simulink model "TosAeb"  (R2021a)
settings: Solver=FixedStepDiscrete  StartTime=0.0  StopTime=inf  FixedStep=0.01  RelTol=1e-3
undefined symbols (not defined in the file or in its folder): ACCModSts, AccSts1, AEB_ObjectMovingOrientation, AEB_ObjectMovingState, CN_BTCActive, CN_CurrentGearR, CN_CurrentGearR_DHT, CN_PTCActive, CN_VDCActive, CN_VSC_EspOff, CN_VSC_FCW_AEB_Error, CN_VSC_FCW_AEB_NoDispay, CN_VSC_FCW_AEB_PerfmDgdtn, CN_VSC_FCW_AEB_TempUnAvlbl, CN_VSC_VehStandstill, CNaccelAvoidMax, CNaccelAvoidMin, CNbrakePedalPress, CNcomfortStrLeftAccelMax, CNcomfortStrLeftAccelMin, CNcomfortStrRightAccelMax, CNcomfortStrRightAccelMin, CNDrvDoorOpen, CNDrvModeEco, CNDrvModeNorm, CNDrvModeOther, CNDrvModeSport, CNDrvSBRreminder, CNNull, CNobjInvalidID, CNPassDoorOpen, CNPassSBRreminder, CNPEBSAccelNegInf, CNPEBSAccelNull, CNPEBSTimeInf, CNPEBSTimeNull, CNSTOPMAX, CNSTOPNULL, CNsubjWidth, CNSwtOFF, CNSwtON, CNTTCMAX, CNTTCNULL, CNVehConfigAEBCycNone, CNVehConfigAEBNone, CNVehConfigAEBPedNone, CNVehConfigFCWNone, CNVehConfigJANone, CollisionSeverity, FALSE, FCWSnvty, JACycObjCalcInfoDefaultParams, JAPedObjCalcInfoDefaultParams, JAVehObjCalcInfoDefaultParams, latCycObjCalcInfoDefaultParams, latPedObjCalcInfoDefaultParams, longObjCalcInfoDefaultParams, ModSts, movingStatus, ObjectType ... (+1112)
libraries: simulink x3031, AEBSFunctionLib x50, Ta_Library x33, AEBSStateMachineLib x23, Mylibrary x12, simulink_extras x7, ObjSelect_lib x3, dspstat3 x2
(part 67 of 102)

== system TosAeb/AEB_Objsel/TA_Processor/AEB_ObjSelect/TargetInfoCheck/Data_precess/TA_EffectCalculate/ValideateObj/ObjPos_Calculate/ObjPos_Calculateelse/InPath_Cal/DetermineInPath ==
  ALat : Inport
  VLat : Inport  Port=2
  PathEstimn : Inport  Port=3
  VLgtRel : Inport  Port=4
  PosnLat : Inport  Port=5
  PosnLgt : Inport  Port=6
  ObjWidth : Inport  Port=7
  HostWidth : Inport  Port=8
  Abs : Abs
  Add1 : Sum  Inputs=+-
  Add2 : Sum  Inputs=+-
  Add3 : Sum
  Add4 : Sum
  Compare To Zero : Subsystem  mask(Compare To Zero){relop=<, OutDataTypeStr=boolean, ZeroCross=on}
      mask prompts: relop "Simulink:masks:Operator_MP"; OutDataTypeStr "Simulink:masks:OutputDataType_MP"; ZeroCross "Simulink:masks:EnableZerocrossingDetection_MP"
      mask help: Simulink:masks:CompareToZero_MD
      mask init: same as TosAeb/AEB_Objsel/TA_Processor/AEB_ObjSelect/TargetInfoCheck/Data_precess/TA_EffectCalculate/Obj_FilterFlgCalculate/Long_ObjFilter/TTR_Calculate/CalculateTTRAndTTM_else/ModifyTTRAtSameSpeedAndAcceleration/Compare To Zero
      mask TypeOptions relop: == | ~= | < | <= | >= | >
      mask TypeOptions OutDataTypeStr: Simulink:masks:uint8 | Simulink:masks:boolean
      mask Callback ZeroCross: sl('zeroCrossEnable', gcbh);
      mask Display: disp([relop,' 0'])
  Constant : Constant  Value=1.8  OutDataTypeStr=single
  Constant2 : Constant  Value=single(0.5)
  Divide4 : Product  Inputs=**
  LimitedSafeDivide : Subsystem
  OppositeSign : Gain  Gain=single(-1)
  PredictHostLateralPositionAtTTR : Subsystem
  PredictObjectLateralPositionAtTTR : Subsystem
  Terminator{1..4} (4 alike) : Terminator
  InPath : Outport
  wires:
  PathEstimn/1 -> PredictHostLateralPositionAtTTR/2(PahEstimn)
  VLgtRel/1 -> OppositeSign/1
  Constant2/1 -> Divide4/2
  OppositeSign/1 -> LimitedSafeDivide/2(Denominator)
  PosnLat/1 -> PredictObjectLateralPositionAtTTR/1(Posn)
  PredictHostLateralPositionAtTTR/3(PredHostHeading) -> Terminator1/1
  Compare To Zero/1(y) -> InPath/1  "InPath"
  PredictObjectLateralPositionAtTTR/2(VPred) -> Terminator3/1
  PredictHostLateralPositionAtTTR/2(PredHostPosnLat) -> Add1/2  "PosnLatPredHost"
  Add4/1 -> Divide4/1  "ObjectAndHostWidth"
  Add1/1 -> Abs/1  "PosnLatPredRel"
  Abs/1 -> Add2/1  "LateralDistance"
  PredictObjectLateralPositionAtTTR/3(APred) -> Terminator4/1
  Divide4/1 -> Add2/2  "HalfObjectAndHostWidth"
  Add2/1 -> Compare To Zero/1(u)  "LatDistanceEdgeToEdge"
  PredictObjectLateralPositionAtTTR/1(PosnPred) -> Add1/1  "PosnLatPredObj"
  PredictHostLateralPositionAtTTR/1(PredHostPosnLgt) -> Terminator2/1
  LimitedSafeDivide/1(LimitedQuotient) -> PredictHostLateralPositionAtTTR/1(PredictionTime), PredictObjectLateralPositionAtTTR/4(Ti)  "SimpleTTR"
  VLat/1 -> PredictObjectLateralPositionAtTTR/2(V)
  ALat/1 -> PredictObjectLateralPositionAtTTR/3(A)
  PosnLgt/1 -> LimitedSafeDivide/1(Numerator)
  ObjWidth/1 -> Add4/1
  HostWidth/1 -> Add3/1
  Add3/1 -> Add4/2  "ObjectAndHostWidth"
  Constant/1 -> Add3/2

== system TosAeb/AEB_Objsel/TA_Processor/AEB_ObjSelect/TargetInfoCheck/Data_precess/TA_EffectCalculate/ValideateObj/ValidObj_PreProcess/If Action Subsystem1/CheckPosition/Subsystem ==
  ObsDx : Inport
  If : If
  Merge : Merge
  dxCalc_if : Subsystem  TreatAsAtomicUnit=on
  dxcalc : Subsystem  TreatAsAtomicUnit=on
  Obsdx : Outport
  wires:
  ObsDx/1 -> [A], If/1
  If/1 -> dxCalc_if/Action
  If/2 -> dxcalc/Action
  [A] -> dxCalc_if/1(ObsDx)
  dxCalc_if/1(Obsdx) -> Merge/1
  dxcalc/1(Obsdx) -> Merge/2
  Merge/1 -> Obsdx/1

== system TosAeb/AEB_Objsel/TA_Processor/AEB_ObjSelect/TargetInfoCheck/Data_precess/TA_EffectCalculate/ValideateObj/ValidObj_PreProcess/If Action Subsystem1/CheckType/Bicycle ==
  TA_ObsType : Inport
  Constant8 : Constant  Value=5  OutDataTypeStr=uint8
  Relational Operator8 : RelationalOperator  Operator===  OutDataTypeStr=boolean  RndMeth=Simplest
  Bicycle_Flg : Outport
  wires:
  TA_ObsType/1 -> Relational Operator8/1
  Constant8/1 -> Relational Operator8/2
  Relational Operator8/1 -> Bicycle_Flg/1

== system TosAeb/AEB_Objsel/TA_Processor/AEB_ObjSelect/TargetInfoCheck/Data_precess/TA_EffectCalculate/ValideateObj/ValidObj_PreProcess/If Action Subsystem1/CheckType/Bus ==
  TA_ObsType : Inport
  Constant6 : Constant  OutDataTypeStr=uint8
  Relational Operator6 : RelationalOperator  Operator===  OutDataTypeStr=boolean  RndMeth=Simplest
  Bus_Flg : Outport
  wires:
  TA_ObsType/1 -> Relational Operator6/1
  Constant6/1 -> Relational Operator6/2
  Relational Operator6/1 -> Bus_Flg/1

== system TosAeb/AEB_Objsel/TA_Processor/AEB_ObjSelect/TargetInfoCheck/Data_precess/TA_EffectCalculate/ValideateObj/ValidObj_PreProcess/If Action Subsystem1/CheckType/Car ==
  TA_ObsType : Inport
  Constant3 : Constant  Value=2  OutDataTypeStr=uint8
  Relational Operator3 : RelationalOperator  Operator===  OutDataTypeStr=boolean  RndMeth=Simplest
  Car_Flg : Outport
  wires:
  TA_ObsType/1 -> Relational Operator3/1
  Constant3/1 -> Relational Operator3/2
  Relational Operator3/1 -> Car_Flg/1

== system TosAeb/AEB_Objsel/TA_Processor/AEB_ObjSelect/TargetInfoCheck/Data_precess/TA_EffectCalculate/ValideateObj/ValidObj_PreProcess/If Action Subsystem1/CheckType/Invalid ==
  TA_ObjType : Inport
  Constant1 : Constant  Value=7  OutDataTypeStr=uint8
  Constant8 : Constant  Value=0  OutDataTypeStr=uint8
  Logical Operator : Logic  Operator=OR  AllPortsSameDT=off  OutDataTypeStr=boolean
  Logical Operator1 : Logic  Operator=NOT  AllPortsSameDT=off  OutDataTypeStr=boolean
  Relational Operator1 : RelationalOperator  Operator===  OutDataTypeStr=boolean  RndMeth=Simplest
  Relational Operator8 : RelationalOperator  Operator===  OutDataTypeStr=boolean  RndMeth=Simplest
  Invalid_Flg : Outport
  wires:
  TA_ObjType/1 -> Relational Operator1/1, Relational Operator8/1
  Constant8/1 -> Relational Operator8/2
  Constant1/1 -> Relational Operator1/2
  Relational Operator8/1 -> Logical Operator/1
  Relational Operator1/1 -> Logical Operator/2
  Logical Operator/1 -> Logical Operator1/1
  Logical Operator1/1 -> Invalid_Flg/1

== system TosAeb/AEB_Objsel/TA_Processor/AEB_ObjSelect/TargetInfoCheck/Data_precess/TA_EffectCalculate/ValideateObj/ValidObj_PreProcess/If Action Subsystem1/CheckType/MotorBike ==
  TA_ObsType : Inport
  Constant7 : Constant  Value=4  OutDataTypeStr=uint8
  Relational Operator7 : RelationalOperator  Operator===  OutDataTypeStr=boolean  RndMeth=Simplest
  MotorBike : Outport
  wires:
  Constant7/1 -> Relational Operator7/2
  Relational Operator7/1 -> MotorBike/1
  TA_ObsType/1 -> Relational Operator7/1

== system TosAeb/AEB_Objsel/TA_Processor/AEB_ObjSelect/TargetInfoCheck/Data_precess/TA_EffectCalculate/ValideateObj/ValidObj_PreProcess/If Action Subsystem1/CheckType/Pedestrain ==
  TA_ObjType : Inport
  Constant8 : Constant  Value=6  OutDataTypeStr=uint8
  Relational Operator8 : RelationalOperator  Operator===  OutDataTypeStr=boolean  RndMeth=Simplest
  Pedestrian_Flg : Outport
  wires:
  Constant8/1 -> Relational Operator8/2
  TA_ObjType/1 -> Relational Operator8/1
  Relational Operator8/1 -> Pedestrian_Flg/1

== system TosAeb/AEB_Objsel/TA_Processor/AEB_ObjSelect/TargetInfoCheck/Data_precess/TA_EffectCalculate/ValideateObj/ValidObj_PreProcess/If Action Subsystem1/CheckType/Truck ==
  TA_ObsType : Inport
  Constant2 : Constant  Value=3  OutDataTypeStr=uint8
  Relational Operator2 : RelationalOperator  Operator===  OutDataTypeStr=boolean  RndMeth=Simplest
  Truck_Flg : Outport
  wires:
  Constant2/1 -> Relational Operator2/2
  TA_ObsType/1 -> Relational Operator2/1
  Relational Operator2/1 -> Truck_Flg/1

== system TosAeb/AEB_Objsel/TA_Processor/PreProcessor/ObjectPrecessor/TAObjInfoCalc/For Each Subsystem/Info_Check1/BasicInfo_check/AccCorrect/Acc_XY/Subsystem ==
  ObsAccY_s : Inport
  Obj_vy : Inport  Port=2
  Add : Sum
  Add2 : Sum  Inputs=+-
  Compare To Constant : simulink/Logic and Bit Operations/Compare To Constant  relop=>=  const=2  OutDataTypeStr=boolean
  Constant : Constant  Value=0.1  OutDataTypeStr=single
  Constant4 : Constant  Value=P_TA_ObjAyLowPassFactor
  Delay : Delay
  Divide : Product  Inputs=*/
  LowPassFilter : Ta_Library/LowPassFilter  SystemSampleTime=-1  Latency=0  AutoFrameSizeCalculation=off
  Switch : Switch  Criteria=u2 ~= 0
  ObsAy_Correct : Outport
  wires:
  Divide/1 -> Add2/2
  ObsAccY_s/1 -> [ObsAccY_s], Add2/1, LowPassFilter/2
  Add2/1 -> Compare To Constant/1
  Obj_vy/1 -> Delay/1, Add/1
  Delay/1 -> Add/2
  Add/1 -> Divide/1
  Constant/1 -> Divide/2
  Compare To Constant/1 -> Switch/2
  Constant4/1 -> LowPassFilter/1
  LowPassFilter/1 -> Switch/1
  [ObsAccY_s] -> Switch/3
  Switch/1 -> ObsAy_Correct/1

== system TosAeb/AEB_Objsel/TA_Processor/PreProcessor/ObjectPrecessor/TAObjInfoCalc/For Each Subsystem/Info_Check1/BasicInfo_check/AccCorrect/Acc_XY/Subsystem1 ==
  ObsAccX_s : Inport
  Obj_vX : Inport  Port=2
  Add : Sum
  Add2 : Sum  Inputs=+-
  Compare To Constant : simulink/Logic and Bit Operations/Compare To Constant  relop=>=  const=2  OutDataTypeStr=boolean
  Constant : Constant  Value=0.1  OutDataTypeStr=single
  Constant4 : Constant  Value=P_TA_ObjAxLowPassFactor
  Delay : Delay
  Divide : Product  Inputs=*/
  LowPassFilter : Ta_Library/LowPassFilter  SystemSampleTime=-1  Latency=0  AutoFrameSizeCalculation=off
  Switch : Switch  Criteria=u2 ~= 0
  ObsAX_Correct : Outport
  wires:
  Switch/1 -> ObsAX_Correct/1
  [ObsAccY_s] -> Switch/3
  LowPassFilter/1 -> Switch/1
  Constant4/1 -> LowPassFilter/1
  Compare To Constant/1 -> Switch/2
  Constant/1 -> Divide/2
  Add/1 -> Divide/1
  Delay/1 -> Add/2
  Obj_vX/1 -> Add/1, Delay/1
  Add2/1 -> Compare To Constant/1
  ObsAccX_s/1 -> LowPassFilter/2, Add2/1, [ObsAccY_s]
  Divide/1 -> Add2/2

== system TosAeb/AEB_Objsel/TA_Processor/PreProcessor/ObjectPrecessor/TAObjInfoCalc/For Each Subsystem/Info_Check1/BasicInfo_check/DxCorrect/dx_charge/Dx_Flag ==
  Fus_ObjVx_Single : Inport
  Veh_AEBFunState : Inport  Port=2
  Fus_ObjDxStd : Inport  Port=3
  Fus_ObjDx_Single : Inport  Port=4
  Compare To Constant : simulink/Logic and Bit Operations/Compare To Constant  relop=>=  const=P_TA_ObjDxRateVarianceThredValue  OutDataTypeStr=boolean
  Compare To Constant1 : simulink/Logic and Bit Operations/Compare To Constant  relop=>=  const=P_TA_ObjDxSTDThredValue  OutDataTypeStr=boolean
  Data Type Conversion : DataTypeConversion
  Logical Operator : Logic  Operator=OR  Inputs=3  AllPortsSameDT=off  OutDataTypeStr=boolean
  Logical Operator1 : Logic  AllPortsSameDT=off  OutDataTypeStr=boolean
  RateVariance_Cal : Ta_Library/RateVariance_Cal  SystemSampleTime=-1  Latency=0  AutoFrameSizeCalculation=off
  dx_AebState : Subsystem
  dx_compare : Subsystem
  Dx_CorrectFlg : Outport
  wires:
  RateVariance_Cal/1 -> Compare To Constant/1
  Fus_ObjDx_Single/1 -> RateVariance_Cal/1, dx_compare/1(Fus_ObjDx_Single)
  dx_AebState/1(AEB_State) -> Logical Operator1/2
  Compare To Constant/1 -> Logical Operator/1
  Veh_AEBFunState/1 -> dx_AebState/1(Veh_AEBFunState)
  Logical Operator/1 -> Logical Operator1/1
  dx_compare/1(DisLong_flg) -> Logical Operator/2
  Fus_ObjVx_Single/1 -> dx_compare/2(Fus_ObjVx_Single)
  Logical Operator1/1 -> Data Type Conversion/1
  Data Type Conversion/1 -> Dx_CorrectFlg/1
  Compare To Constant1/1 -> Logical Operator/3
  Fus_ObjDxStd/1 -> Compare To Constant1/1

== system TosAeb/AEB_Objsel/TA_Processor/PreProcessor/ObjectPrecessor/TAObjInfoCalc/For Each Subsystem/Info_Check1/BasicInfo_check/DxCorrect/dx_charge/dx_change ==
  Dx_CorrectFlg : Inport
  Fus_ObjDx_Single : Inport  Port=2
  Constant : Constant  Value=P_TA_ObjDxLowPassFactor
  Constant1 : Constant  Value=0  OutDataTypeStr=boolean
  LowPassFilter : Ta_Library/LowPassFilter  SystemSampleTime=-1  Latency=0  AutoFrameSizeCalculation=off
  Switch : Switch  Criteria=u2 ~= 0
  Terminator_1 : Terminator
  Fus_ObjDx : Outport
  wires:
  LowPassFilter/1 -> Switch/1
  Switch/1 -> Fus_ObjDx/1
  Constant/1 -> LowPassFilter/1
  Constant1/1 -> Switch/2
  Dx_CorrectFlg/1 -> Terminator_1/1
  Fus_ObjDx_Single/1 -> Switch/3, LowPassFilter/2

== system TosAeb/AEB_Objsel/TA_Processor/PreProcessor/ObjectPrecessor/TAObjInfoCalc/For Each Subsystem/Info_Check1/BasicInfo_check/DyCorrect/dy_charge/Subsystem2 ==
  Fus_ObjVy_Single : Inport
  Veh_AEBFunState : Inport  Port=2
  Fus_ObsDyStd : Inport  Port=3
  Fus_ObjDy_Single : Inport  Port=4
  Compare To Constant : simulink/Logic and Bit Operations/Compare To Constant  relop=>=  const=P_TA_ObjDyRateVarianceThredValue  OutDataTypeStr=boolean
  Compare To Constant1 : simulink/Logic and Bit Operations/Compare To Constant  relop=>=  const=P_TA_ObjDySTDThredValue  OutDataTypeStr=boolean
  Data Type Conversion : DataTypeConversion
  Logical Operator : Logic  Operator=OR  Inputs=3  AllPortsSameDT=off  OutDataTypeStr=boolean
  Logical Operator1 : Logic  AllPortsSameDT=off  OutDataTypeStr=boolean
  RateVariance_Cal : Ta_Library/RateVariance_Cal  SystemSampleTime=-1  Latency=0  AutoFrameSizeCalculation=off
  Subsystem1 : Subsystem
  charge : Subsystem
  Dy_CorrectFlg : Outport
  wires:
  Data Type Conversion/1 -> Dy_CorrectFlg/1
  Logical Operator1/1 -> Data Type Conversion/1
  Fus_ObjVy_Single/1 -> charge/2(Fus_ObjVy_Single)
  charge/1(DisLat_flg) -> Logical Operator/2
  Logical Operator/1 -> Logical Operator1/1
  Veh_AEBFunState/1 -> Subsystem1/1(Veh_AEBFunState)
  Compare To Constant/1 -> Logical Operator/1
  Subsystem1/1(AEB_State) -> Logical Operator1/2
  Fus_ObjDy_Single/1 -> charge/1(Fus_ObjDy_Single), RateVariance_Cal/1
  RateVariance_Cal/1 -> Compare To Constant/1
  Compare To Constant1/1 -> Logical Operator/3
  Fus_ObsDyStd/1 -> Compare To Constant1/1

== system TosAeb/AEB_Objsel/TA_Processor/PreProcessor/ObjectPrecessor/TAObjInfoCalc/For Each Subsystem/Info_Check1/BasicInfo_check/DyCorrect/dy_charge/dy_change ==
  Dy_CorrectFlg : Inport
  Fus_ObjDy_Single : Inport  Port=2
  Constant : Constant  Value=P_TA_ObjDyLowPassFactor
  Constant1 : Constant  Value=0  OutDataTypeStr=boolean
  LowPassFilter : Ta_Library/LowPassFilter  SystemSampleTime=-1  Latency=0  AutoFrameSizeCalculation=off
  Scope : Scope  NumInputPorts=3
  Switch : Switch  Criteria=u2 ~= 0
  Terminator : Terminator
  Fus_ObjDy : Outport
  wires:
  Switch/1 -> Scope/3, Fus_ObjDy/1
  LowPassFilter/1 -> Switch/1, Scope/2
  Fus_ObjDy_Single/1 -> LowPassFilter/2, Scope/1, Switch/3
  Constant/1 -> LowPassFilter/1
  Constant1/1 -> Switch/2
  Dy_CorrectFlg/1 -> Terminator/1

== system TosAeb/AEB_Objsel/TA_Processor/PreProcessor/ObjectPrecessor/TAObjInfoCalc/For Each Subsystem/Info_Check1/BasicInfo_check/HeadAngle/If Action Subsystem1/HeadAngle_c ==
  ID_Switch : Inport
  ObsAngle_s : Inport  Port=2
  ObsAngleSTD_s : Inport  Port=3
  Obj_VxCr : Inport  Port=4
  Obj_VyCr : Inport  Port=5
  Add : Sum  Inputs=+-
  Add1 : Sum
  Compare To Constant : simulink/Logic and Bit Operations/Compare To Constant  relop=<  const=P_TA_ObjHeadAngleDiffThred  OutDataTypeStr=boolean
  Compare To Constant1 : simulink/Logic and Bit Operations/Compare To Constant  relop=<  const=P_TA_ObjHeadAngleSTDThredValue  OutDataTypeStr=boolean
  Constant : Constant  Value=P_TA_ObjHeadAngleLowPassFactor
  Constant1 : Constant  Value=0.5  OutDataTypeStr=single
  Constant2 : Constant  Value=0  OutDataTypeStr=single
  Logical Operator : Logic  AllPortsSameDT=off  OutDataTypeStr=boolean
  LowPassFilter : Ta_Library/LowPassFilter  SystemSampleTime=-1  Latency=0  AutoFrameSizeCalculation=off
  Product : Product
  Subsystem2 : Subsystem
  Switch : Switch  Criteria=u2 > Threshold
  Switch1 : Switch  Criteria=u2 > Threshold
  Fus_ObjHeadAngle : Outport
  wires:
  Obj_VxCr/1 -> Subsystem2/2(Obj_Vx)
  Obj_VyCr/1 -> Subsystem2/1(Obj_vy)
  Subsystem2/1(Obj_Head) -> Add1/1, Add/2
  ObsAngle_s/1 -> [ObsAngle_s], Add/1
  Add/1 -> Compare To Constant/1
  ObsAngleSTD_s/1 -> Compare To Constant1/1
  Compare To Constant1/1 -> Logical Operator/1
  Compare To Constant/1 -> Logical Operator/2
  Logical Operator/1 -> Switch/2
  [ObsAngle_s] -> Switch/1
  [ObsAngle_s] -> Add1/2
  Add1/1 -> Product/1
  Constant/1 -> LowPassFilter/1
  Constant1/1 -> Product/2
  Product/1 -> LowPassFilter/2
  LowPassFilter/1 -> Switch/3
  Switch/1 -> Switch1/3
  ID_Switch/1 -> Switch1/2
  Switch1/1 -> Fus_ObjHeadAngle/1
  Constant2/1 -> Switch1/1

== system TosAeb/AEB_Objsel/TA_Processor/PreProcessor/ObjectPrecessor/TAObjInfoCalc/For Each Subsystem/Info_Check1/BasicInfo_check/HeadAngle/If Action Subsystem1/Type_class ==
  Fus_Type : Inport
  Compare To Constant : simulink/Logic and Bit Operations/Compare To Constant  relop=<  const=1  OutDataTypeStr=boolean
  Compare To Constant1 : simulink/Logic and Bit Operations/Compare To Constant  relop=>  const=10  OutDataTypeStr=boolean
  Data Type Conversion : DataTypeConversion
  Logical Operator : Logic  Operator=OR  AllPortsSameDT=off  OutDataTypeStr=boolean
  tyoe_enable : Outport
  wires:
  Fus_Type/1 -> Compare To Constant1/1, Compare To Constant/1
  Compare To Constant/1 -> Logical Operator/1
  Compare To Constant1/1 -> Logical Operator/2
  Logical Operator/1 -> Data Type Conversion/1
  Data Type Conversion/1 -> tyoe_enable/1

== system TosAeb/AEB_Objsel/TA_Processor/PreProcessor/ObjectPrecessor/TAObjInfoCalc/For Each Subsystem/Info_Check1/BasicInfo_check/QuaCorrect/quality_recrectionelse/Quality_else ==
  Fus_TargetQuality : Inport
  dy_flg : Inport  Port=2
  dx_flg : Inport  Port=3
  vx_flg : Inport  Port=4
  vy_flg : Inport  Port=5
  Action Port : ActionPort
  Factor_Cal : Subsystem
  Quality_Out : Subsystem
  Fus_ObjQuality : Outport
  wires:
  vx_flg/1 -> Factor_Cal/3(vx_flg)
  Quality_Out/1(Fus_ObjQuality) -> Fus_ObjQuality/1
  dy_flg/1 -> Factor_Cal/1(dy_flg)
  Factor_Cal/1(factor) -> Quality_Out/2(factor)
  dx_flg/1 -> Factor_Cal/2(dx_flg)
  vy_flg/1 -> Factor_Cal/4(vy_flg)
  Fus_TargetQuality/1 -> Quality_Out/1(Fus_TargetQuality)

== system TosAeb/AEB_Objsel/TA_Processor/PreProcessor/ObjectPrecessor/TAObjInfoCalc/For Each Subsystem/Info_Check1/BasicInfo_check/QuaCorrect/quality_recrectionelse/Quality_if ==
  Fus_TargetQuality : Inport
  Action Port : ActionPort
  Signal Conversion : SignalConversion  OverrideOpt=off
  Fus_ObjQuality : Outport
  wires:
  Signal Conversion/1 -> Fus_ObjQuality/1
  Fus_TargetQuality/1 -> Signal Conversion/1

== system TosAeb/AEB_Objsel/TA_Processor/PreProcessor/ObjectPrecessor/TAObjInfoCalc/For Each Subsystem/Info_Check1/BasicInfo_check/QuaCorrect/quality_recrectionelse/Subsystem1 ==
  Veh_AEBFunState : Inport
  Compare To Constant : simulink/Logic and Bit Operations/Compare To Constant  relop===  const=1  OutDataTypeStr=boolean
  Data Type Conversion : DataTypeConversion
  AEB_State : Outport
  wires:
  Data Type Conversion/1 -> AEB_State/1
  Compare To Constant/1 -> Data Type Conversion/1
  Veh_AEBFunState/1 -> Compare To Constant/1

== system TosAeb/AEB_Objsel/TA_Processor/PreProcessor/ObjectPrecessor/TAObjInfoCalc/For Each Subsystem/Info_Check1/BasicInfo_check/VxCorrect/Subsystem3/Subsystem1 ==
  Vx_CorrectFlg : Inport
  Fus_ObjVx_Single : Inport  Port=2
  Constant : Constant  Value=P_TA_ObjVxLowPassFactor
  LowPassFilter : Ta_Library/LowPassFilter  SystemSampleTime=-1  Latency=0  AutoFrameSizeCalculation=off
  Switch : Switch  Criteria=u2 ~= 0
  Fus_ObjVx : Outport
  wires:
  Switch/1 -> Fus_ObjVx/1
  Fus_ObjVx_Single/1 -> Switch/3, LowPassFilter/2
  LowPassFilter/1 -> Switch/1
  Vx_CorrectFlg/1 -> Switch/2
  Constant/1 -> LowPassFilter/1

== system TosAeb/AEB_Objsel/TA_Processor/PreProcessor/ObjectPrecessor/TAObjInfoCalc/For Each Subsystem/Info_Check1/BasicInfo_check/VxCorrect/Subsystem3/Subsystem2 ==
  Fus_ObjDx_Single : Inport
  Veh_AEBFunState : Inport  Port=2
  Fus_ObjVxStd : Inport  Port=3
  Fus_ObjVx_Single : Inport  Port=4
  Compare To Constant : simulink/Logic and Bit Operations/Compare To Constant  relop=>=  const=P_TA_ObjVxVarianceThredValue  OutDataTypeStr=boolean
  Compare To Constant1 : simulink/Logic and Bit Operations/Compare To Constant  relop=>=  const=P_TA_ObjVxSTDThredValue  OutDataTypeStr=boolean
  Data Type Conversion : DataTypeConversion
  Logical Operator : Logic  Operator=OR  Inputs=3  AllPortsSameDT=off  OutDataTypeStr=boolean
  Logical Operator1 : Logic  AllPortsSameDT=off  OutDataTypeStr=boolean
  Subsystem : Subsystem
  Subsystem1 : Subsystem
  Variance_Cal : Ta_Library/Variance_Cal  SystemSampleTime=-1  Latency=0  AutoFrameSizeCalculation=off
  Vx_CorrectFlg : Outport
  wires:
  Variance_Cal/1 -> Compare To Constant/1
  Data Type Conversion/1 -> Vx_CorrectFlg/1
  Logical Operator1/1 -> Data Type Conversion/1
  Fus_ObjDx_Single/1 -> Subsystem/2(Fus_ObjVx_Single)
  Subsystem/1(DisLong_flg) -> Logical Operator/2
  Logical Operator/1 -> Logical Operator1/1
  Veh_AEBFunState/1 -> Subsystem1/1(Veh_AEBFunState)
  Compare To Constant/1 -> Logical Operator/1
  Subsystem1/1(AEB_State) -> Logical Operator1/2
  Fus_ObjVx_Single/1 -> Subsystem/1(Fus_ObjDx_Single), Variance_Cal/1
  Fus_ObjVxStd/1 -> Compare To Constant1/1
  Compare To Constant1/1 -> Logical Operator/3

== system TosAeb/AEB_Objsel/TA_Processor/PreProcessor/ObjectPrecessor/TAObjInfoCalc/For Each Subsystem/Info_Check1/BasicInfo_check/VyCorrect/Subsystem3/Subsystem1 ==
  Vy_CorrectFlg : Inport
  State_flg : Inport  Port=2
  Fus_ObjVy_Single : Inport  Port=3
  Constant : Constant  Value=P_TA_ObjVyLowPassFactor
  Constant1 : Constant  Value=0  OutDataTypeStr=single
  LowPassFilter : Ta_Library/LowPassFilter  SystemSampleTime=-1  Latency=0  AutoFrameSizeCalculation=off
  Switch : Switch  Criteria=u2 ~= 0
  Switch2 : Switch  Criteria=u2 ~= 0
  Fus_ObjVy : Outport
  wires:
  Switch2/1 -> Fus_ObjVy/1
  Constant1/1 -> Switch2/1
  State_flg/1 -> Switch2/2
  Vy_CorrectFlg/1 -> Switch/2
  Fus_ObjVy_Single/1 -> LowPassFilter/2, Switch/3
  Switch/1 -> Switch2/3
  LowPassFilter/1 -> Switch/1
  Constant/1 -> LowPassFilter/1

== system TosAeb/AEB_Objsel/TA_Processor/PreProcessor/ObjectPrecessor/TAObjInfoCalc/For Each Subsystem/Info_Check1/BasicInfo_check/VyCorrect/Subsystem3/Subsystem2 ==
  Fus_ObjDy_Single : Inport
  Veh_AEBFunState : Inport  Port=2
  Fus_TargetState : Inport  Port=3
  Fus_ObsVyStd : Inport  Port=4
  Veh_YawRate : Inport  Port=5
  Fus_ObjVy_Single : Inport  Port=6
  Compare To Constant : simulink/Logic and Bit Operations/Compare To Constant  relop=>=  const=P_TA_ObjVyVarianceThredValue  OutDataTypeStr=boolean
  Compare To Constant1 : simulink/Logic and Bit Operations/Compare To Constant  relop=>=  const=P_TA_ObjVySTDThredValue  OutDataTypeStr=boolean
  Data Type Conversion : DataTypeConversion
  Logical Operator : Logic  Operator=OR  Inputs=3  AllPortsSameDT=off  OutDataTypeStr=boolean
  Logical Operator1 : Logic  AllPortsSameDT=off  OutDataTypeStr=boolean
  Subsystem : Subsystem
  Subsystem1 : Subsystem
  Subsystem2 : Subsystem
  Variance_Cal : Ta_Library/Variance_Cal  SystemSampleTime=-1  Latency=0  AutoFrameSizeCalculation=off
  Vy_CorrectFlg : Outport
  State_flg : Outport  Port=2
  wires:
  Subsystem2/1(State_flg) -> State_flg/1
  Fus_TargetState/1 -> Subsystem2/1(Fus_TargetState)
  Fus_ObjVy_Single/1 -> Subsystem/1(Fus_ObjDy_Single), Variance_Cal/1
  Subsystem1/1(AEB_State) -> Logical Operator1/2
  Compare To Constant/1 -> Logical Operator/1
  Veh_AEBFunState/1 -> Subsystem1/1(Veh_AEBFunState)
  Logical Operator/1 -> Logical Operator1/1
  Subsystem/1(DisLat_flg) -> Logical Operator/2
  Fus_ObjDy_Single/1 -> Subsystem/2(Fus_ObjVy_Single)
  Logical Operator1/1 -> Data Type Conversion/1
  Data Type Conversion/1 -> Vy_CorrectFlg/1
  Variance_Cal/1 -> Compare To Constant/1
  Compare To Constant1/1 -> Logical Operator/3
  Fus_ObsVyStd/1 -> Compare To Constant1/1
  Veh_YawRate/1 -> Subsystem2/2(Veh_YawRate)

== system TosAeb/AEB_Objsel/TA_Processor/PreProcessor/ObjectPrecessor/TAObjInfoCalc/For Each Subsystem/Info_Check1/Basic_Info_check/AccCorrect/Acc_XY/Subsystem ==
  ObsAccY_s : Inport
  Obj_vy : Inport  Port=2
  Add : Sum
  Add2 : Sum  Inputs=+-
  Compare To Constant : simulink/Logic and Bit Operations/Compare To Constant  relop=>=  const=2  OutDataTypeStr=boolean
  Constant : Constant  Value=0.04  OutDataTypeStr=single
  Constant4 : Constant  Value=P_TA_ObjAyLowPassFactor
  Delay : Delay
  Divide : Product  Inputs=*/
  LowPassFilter : Ta_Library/LowPassFilter  SystemSampleTime=-1  VariantActivationTime=update diagram  Latency=0  AutoFrameSizeCalculation=off
  Switch : Switch  Criteria=u2 ~= 0
  ObsAy_Correct : Outport
  wires:
  Divide/1 -> Add2/2
  ObsAccY_s/1 -> [ObsAccY_s], Add2/1, LowPassFilter/2
  Add2/1 -> Compare To Constant/1
  Obj_vy/1 -> Delay/1, Add/1
  Add/1 -> Divide/1
  Constant/1 -> Divide/2
  Compare To Constant/1 -> Switch/2
  Constant4/1 -> LowPassFilter/1
  LowPassFilter/1 -> Switch/1
  [ObsAccY_s] -> Switch/3
  Switch/1 -> ObsAy_Correct/1
  Delay/1 -> Add/2

== system TosAeb/AEB_Objsel/TA_Processor/PreProcessor/ObjectPrecessor/TAObjInfoCalc/For Each Subsystem/Info_Check1/Basic_Info_check/AccCorrect/Acc_XY/Subsystem1 ==
  ObsAccX_s : Inport
  Obj_vX : Inport  Port=2
  Add : Sum
  Add2 : Sum  Inputs=+-
  Compare To Constant : simulink/Logic and Bit Operations/Compare To Constant  relop=>=  const=2  OutDataTypeStr=boolean
  Constant : Constant  Value=0.04  OutDataTypeStr=single
  Constant4 : Constant  Value=P_TA_ObjAxLowPassFactor
  Delay : Delay
  Divide : Product  Inputs=*/
  LowPassFilter : Ta_Library/LowPassFilter  SystemSampleTime=-1  VariantActivationTime=update diagram  Latency=0  AutoFrameSizeCalculation=off
  Switch : Switch  Criteria=u2 ~= 0
  ObsAX_Correct : Outport
  wires:
  Switch/1 -> ObsAX_Correct/1
  [ObsAccY_s] -> Switch/3
  LowPassFilter/1 -> Switch/1
  Constant4/1 -> LowPassFilter/1
  Compare To Constant/1 -> Switch/2
  Constant/1 -> Divide/2
  Add/1 -> Divide/1
  Obj_vX/1 -> Delay/1, Add/1
  Add2/1 -> Compare To Constant/1
  ObsAccX_s/1 -> LowPassFilter/2, Add2/1, [ObsAccY_s]
  Divide/1 -> Add2/2
  Delay/1 -> Add/2

== system TosAeb/AEB_Objsel/TA_Processor/PreProcessor/ObjectPrecessor/TAObjInfoCalc/For Each Subsystem/Info_Check1/Basic_Info_check/DxCorrect/dx_charge/Dx_Flag ==
  Fus_ObjVx_Single : Inport
  Veh_AEBFunState : Inport  Port=2
  Fus_ObjDxStd : Inport  Port=3
  Fus_ObjDx_Single : Inport  Port=4
  Compare To Constant : simulink/Logic and Bit Operations/Compare To Constant  relop=>=  const=P_TA_ObjDxRateVarianceThredValue  OutDataTypeStr=boolean
  Compare To Constant1 : simulink/Logic and Bit Operations/Compare To Constant  relop=>=  const=P_TA_ObjDxSTDThredValue  OutDataTypeStr=boolean
  Data Type Conversion : DataTypeConversion
  Logical Operator : Logic  Operator=OR  Inputs=3  AllPortsSameDT=off  OutDataTypeStr=boolean
  Logical Operator1 : Logic  AllPortsSameDT=off  OutDataTypeStr=boolean
  RateVariance_Cal1 : Ta_Library/RateVariance_Cal  SystemSampleTime=-1  VariantActivationTime=update diagram  Latency=0  AutoFrameSizeCalculation=off
  dx_AebState : Subsystem
  dx_compare : Subsystem
  Dx_CorrectFlg : Outport
  wires:
  RateVariance_Cal1/1 -> Compare To Constant/1
  Fus_ObjDx_Single/1 -> RateVariance_Cal1/1, dx_compare/1(Fus_ObjDx_Single)
  dx_AebState/1(AEB_State) -> Logical Operator1/2
  Compare To Constant/1 -> Logical Operator/1
  Veh_AEBFunState/1 -> dx_AebState/1(Veh_AEBFunState)
  Logical Operator/1 -> Logical Operator1/1
  dx_compare/1(DisLong_flg) -> Logical Operator/2
  Logical Operator1/1 -> Data Type Conversion/1
  Data Type Conversion/1 -> Dx_CorrectFlg/1
  Compare To Constant1/1 -> Logical Operator/3
  Fus_ObjDxStd/1 -> Compare To Constant1/1
  Fus_ObjVx_Single/1 -> dx_compare/2(Fus_ObjVx_Single)

== system TosAeb/AEB_Objsel/TA_Processor/PreProcessor/ObjectPrecessor/TAObjInfoCalc/For Each Subsystem/Info_Check1/Basic_Info_check/DxCorrect/dx_charge/dx_change ==
  Dx_CorrectFlg : Inport
  Fus_ObjDx_Single : Inport  Port=2
  Constant : Constant  Value=P_TA_ObjDxLowPassFactor
  Constant1 : Constant  Value=0  OutDataTypeStr=boolean
  LowPassFilter : Ta_Library/LowPassFilter  SystemSampleTime=-1  VariantActivationTime=update diagram  Latency=0  AutoFrameSizeCalculation=off
  Switch : Switch  Criteria=u2 ~= 0
  Terminator_1 : Terminator
  Fus_ObjDx : Outport
  wires:
  LowPassFilter/1 -> Switch/1
  Switch/1 -> Fus_ObjDx/1
  Constant/1 -> LowPassFilter/1
  Constant1/1 -> Switch/2
  Dx_CorrectFlg/1 -> Terminator_1/1
  Fus_ObjDx_Single/1 -> Switch/3, LowPassFilter/2

== system TosAeb/AEB_Objsel/TA_Processor/PreProcessor/ObjectPrecessor/TAObjInfoCalc/For Each Subsystem/Info_Check1/Basic_Info_check/DxCorrect1/dx_charge/Dx_Flag ==
  Fus_ObjVx_Single : Inport
  Veh_AEBFunState : Inport  Port=2
  Fus_ObjDxStd : Inport  Port=3
  Fus_ObjDx_Single : Inport  Port=4
  Compare To Constant : simulink/Logic and Bit Operations/Compare To Constant  relop=>=  const=P_TA_ObjDxRateVarianceThredValue  OutDataTypeStr=boolean
  Compare To Constant1 : simulink/Logic and Bit Operations/Compare To Constant  relop=>=  const=P_TA_ObjDxSTDThredValue  OutDataTypeStr=boolean
  Data Type Conversion : DataTypeConversion
  Logical Operator : Logic  Operator=OR  Inputs=3  AllPortsSameDT=off  OutDataTypeStr=boolean
  Logical Operator1 : Logic  AllPortsSameDT=off  OutDataTypeStr=boolean
  RateVariance_Cal1 : Ta_Library/RateVariance_Cal  SystemSampleTime=-1  Latency=0  AutoFrameSizeCalculation=off
  dx_AebState : Subsystem
  dx_compare : Subsystem
  Dx_CorrectFlg : Outport
  wires:
  Fus_ObjVx_Single/1 -> dx_compare/2(Fus_ObjVx_Single)
  Fus_ObjDxStd/1 -> Compare To Constant1/1
  Compare To Constant1/1 -> Logical Operator/3
  Data Type Conversion/1 -> Dx_CorrectFlg/1
  Logical Operator1/1 -> Data Type Conversion/1
  dx_compare/1(DisLong_flg) -> Logical Operator/2
  Logical Operator/1 -> Logical Operator1/1
  Veh_AEBFunState/1 -> dx_AebState/1(Veh_AEBFunState)
  Compare To Constant/1 -> Logical Operator/1
  dx_AebState/1(AEB_State) -> Logical Operator1/2
  Fus_ObjDx_Single/1 -> dx_compare/1(Fus_ObjDx_Single), RateVariance_Cal1/1
  RateVariance_Cal1/1 -> Compare To Constant/1

== system TosAeb/AEB_Objsel/TA_Processor/PreProcessor/ObjectPrecessor/TAObjInfoCalc/For Each Subsystem/Info_Check1/Basic_Info_check/DxCorrect1/dx_charge/dx_change ==
  Dx_CorrectFlg : Inport
  Fus_ObjDx_Single : Inport  Port=2
  Constant : Constant  Value=P_TA_ObjDxLowPassFactor
  Constant1 : Constant  Value=0  OutDataTypeStr=boolean
  LowPassFilter : Ta_Library/LowPassFilter  SystemSampleTime=-1  Latency=0  AutoFrameSizeCalculation=off
  Switch : Switch  Criteria=u2 ~= 0
  Terminator_1 : Terminator
  Fus_ObjDx : Outport
  wires:
  Fus_ObjDx_Single/1 -> LowPassFilter/2, Switch/3
  Dx_CorrectFlg/1 -> Terminator_1/1
  Constant1/1 -> Switch/2
  Constant/1 -> LowPassFilter/1
  Switch/1 -> Fus_ObjDx/1
  LowPassFilter/1 -> Switch/1

== system TosAeb/AEB_Objsel/TA_Processor/PreProcessor/ObjectPrecessor/TAObjInfoCalc/For Each Subsystem/Info_Check1/Basic_Info_check/DyCorrect/dy_charge/Subsystem2 ==
  Fus_ObjVy_Single : Inport
  Veh_AEBFunState : Inport  Port=2
  Fus_ObsDyStd : Inport  Port=3
  Fus_ObjDy_Single : Inport  Port=4
  Compare To Constant : simulink/Logic and Bit Operations/Compare To Constant  relop=>=  const=P_TA_ObjDyRateVarianceThredValue  OutDataTypeStr=boolean
  Compare To Constant1 : simulink/Logic and Bit Operations/Compare To Constant  relop=>=  const=P_TA_ObjDySTDThredValue  OutDataTypeStr=boolean
  Data Type Conversion : DataTypeConversion
  Logical Operator : Logic  Operator=OR  Inputs=3  AllPortsSameDT=off  OutDataTypeStr=boolean
  Logical Operator1 : Logic  AllPortsSameDT=off  OutDataTypeStr=boolean
  Subsystem1 : Subsystem
  Variance_Cal : Ta_Library/Variance_Cal  SystemSampleTime=-1  VariantActivationTime=update diagram  Latency=0  AutoFrameSizeCalculation=off
  charge : Subsystem
  Dy_CorrectFlg : Outport
  wires:
  Data Type Conversion/1 -> Dy_CorrectFlg/1
  Logical Operator1/1 -> Data Type Conversion/1
  Fus_ObjVy_Single/1 -> charge/2(Fus_ObjVy_Single)
  charge/1(DisLat_flg) -> Logical Operator/2
  Logical Operator/1 -> Logical Operator1/1
  Veh_AEBFunState/1 -> Subsystem1/1(Veh_AEBFunState)
  Compare To Constant/1 -> Logical Operator/1
  Subsystem1/1(AEB_State) -> Logical Operator1/2
  Fus_ObjDy_Single/1 -> Variance_Cal/1, charge/1(Fus_ObjDy_Single)
  Variance_Cal/1 -> Compare To Constant/1
  Compare To Constant1/1 -> Logical Operator/3
  Fus_ObsDyStd/1 -> Compare To Constant1/1

== system TosAeb/AEB_Objsel/TA_Processor/PreProcessor/ObjectPrecessor/TAObjInfoCalc/For Each Subsystem/Info_Check1/Basic_Info_check/DyCorrect/dy_charge/dy_change ==
  Dy_CorrectFlg : Inport
  Fus_ObjDy_Single : Inport  Port=2
  Constant : Constant  Value=P_TA_ObjDyLowPassFactor
  Constant1 : Constant  Value=0  OutDataTypeStr=boolean
  LowPassFilter : Ta_Library/LowPassFilter  SystemSampleTime=-1  VariantActivationTime=update diagram  Latency=0  AutoFrameSizeCalculation=off
  Switch : Switch  Criteria=u2 ~= 0
  Terminator : Terminator
  Fus_ObjDy : Outport
  wires:
  Switch/1 -> Fus_ObjDy/1
  LowPassFilter/1 -> Switch/1
  Fus_ObjDy_Single/1 -> LowPassFilter/2, Switch/3
  Constant/1 -> LowPassFilter/1
  Constant1/1 -> Switch/2
  Dy_CorrectFlg/1 -> Terminator/1

== system TosAeb/AEB_Objsel/TA_Processor/PreProcessor/ObjectPrecessor/TAObjInfoCalc/For Each Subsystem/Info_Check1/Basic_Info_check/HeadAngle/If Action Subsystem1/HeadAngle_c ==
  ID_Switch : Inport
  ObsAngle_s : Inport  Port=2
  ObsAngleSTD_s : Inport  Port=3
  Obj_VxCr : Inport  Port=4
  Obj_VyCr : Inport  Port=5
  Add : Sum  Inputs=+-
  Add1 : Sum
  Compare To Constant : simulink/Logic and Bit Operations/Compare To Constant  relop=<  const=P_TA_ObjHeadAngleDiffThred  OutDataTypeStr=boolean
  Compare To Constant1 : simulink/Logic and Bit Operations/Compare To Constant  relop=<  const=P_TA_ObjHeadAngleSTDThredValue  OutDataTypeStr=boolean
  Constant : Constant  Value=P_TA_ObjHeadAngleLowPassFactor
  Constant1 : Constant  Value=0.5  OutDataTypeStr=single
  Constant2 : Constant  Value=0  OutDataTypeStr=single
  Logical Operator : Logic  AllPortsSameDT=off  OutDataTypeStr=boolean
  LowPassFilter : Ta_Library/LowPassFilter  SystemSampleTime=-1  VariantActivationTime=update diagram  Latency=0  AutoFrameSizeCalculation=off
  Product : Product
  Subsystem2 : Subsystem
  Switch : Switch  Criteria=u2 > Threshold
  Switch1 : Switch  Criteria=u2 ~= 0
  Fus_ObjHeadAngle : Outport
  wires:
  Obj_VxCr/1 -> Subsystem2/2(Obj_Vx)
  Obj_VyCr/1 -> Subsystem2/1(Obj_vy)
  Subsystem2/1(Obj_Head) -> Add1/1, Add/2
  ObsAngle_s/1 -> [ObsAngle_s], Add/1
  Add/1 -> Compare To Constant/1
  ObsAngleSTD_s/1 -> Compare To Constant1/1
  Compare To Constant1/1 -> Logical Operator/1
  Compare To Constant/1 -> Logical Operator/2
  Logical Operator/1 -> Switch/2
  [ObsAngle_s] -> Switch/1
  [ObsAngle_s] -> Add1/2
  Add1/1 -> Product/1
  Constant/1 -> LowPassFilter/1
  Constant1/1 -> Product/2
  Product/1 -> LowPassFilter/2
  LowPassFilter/1 -> Switch/3
  Switch/1 -> Switch1/3
  ID_Switch/1 -> Switch1/2
  Switch1/1 -> Fus_ObjHeadAngle/1
  Constant2/1 -> Switch1/1

== system TosAeb/AEB_Objsel/TA_Processor/PreProcessor/ObjectPrecessor/TAObjInfoCalc/For Each Subsystem/Info_Check1/Basic_Info_check/HeadAngle/If Action Subsystem1/Type_class ==
  Fus_Type : Inport
  Compare To Constant : simulink/Logic and Bit Operations/Compare To Constant  relop=<  const=1  OutDataTypeStr=boolean
  Compare To Constant1 : simulink/Logic and Bit Operations/Compare To Constant  relop=>  const=10  OutDataTypeStr=boolean
  Data Type Conversion : DataTypeConversion
  Logical Operator : Logic  Operator=OR  AllPortsSameDT=off  OutDataTypeStr=boolean
  tyoe_enable : Outport
  wires:
  Fus_Type/1 -> Compare To Constant1/1, Compare To Constant/1
  Compare To Constant/1 -> Logical Operator/1
  Compare To Constant1/1 -> Logical Operator/2
  Logical Operator/1 -> Data Type Conversion/1
  Data Type Conversion/1 -> tyoe_enable/1

== system TosAeb/AEB_Objsel/TA_Processor/PreProcessor/ObjectPrecessor/TAObjInfoCalc/For Each Subsystem/Info_Check1/Basic_Info_check/QuaCorrect/quality_recrectionelse/Quality_else ==
  Fus_TargetQuality : Inport
  dy_flg : Inport  Port=2
  dx_flg : Inport  Port=3
  vx_flg : Inport  Port=4
  vy_flg : Inport  Port=5
  Action Port : ActionPort  ActionPortLabel=else
  Factor_Cal : Subsystem
  Quality_Out : Subsystem
  Fus_ObjQuality : Outport
  wires:
  vx_flg/1 -> Factor_Cal/3(vx_flg)
  Quality_Out/1(Fus_ObjQuality) -> Fus_ObjQuality/1
  dy_flg/1 -> Factor_Cal/1(dy_flg)
  Factor_Cal/1(factor) -> Quality_Out/2(factor)
  dx_flg/1 -> Factor_Cal/2(dx_flg)
  vy_flg/1 -> Factor_Cal/4(vy_flg)
  Fus_TargetQuality/1 -> Quality_Out/1(Fus_TargetQuality)

== system TosAeb/AEB_Objsel/TA_Processor/PreProcessor/ObjectPrecessor/TAObjInfoCalc/For Each Subsystem/Info_Check1/Basic_Info_check/QuaCorrect/quality_recrectionelse/Quality_if ==
  Fus_TargetQuality : Inport
  Action Port : ActionPort  ActionPortLabel=if(u1 > 0)
  Signal Conversion : SignalConversion  OverrideOpt=off
  Fus_ObjQuality : Outport
  wires:
  Signal Conversion/1 -> Fus_ObjQuality/1
  Fus_TargetQuality/1 -> Signal Conversion/1

== system TosAeb/AEB_Objsel/TA_Processor/PreProcessor/ObjectPrecessor/TAObjInfoCalc/For Each Subsystem/Info_Check1/Basic_Info_check/QuaCorrect/quality_recrectionelse/Subsystem1 ==
  Veh_AEBFunState : Inport
  Compare To Constant : simulink/Logic and Bit Operations/Compare To Constant  relop===  const=1  OutDataTypeStr=boolean
  Data Type Conversion : DataTypeConversion
  AEB_State : Outport
  wires:
  Data Type Conversion/1 -> AEB_State/1
  Compare To Constant/1 -> Data Type Conversion/1
  Veh_AEBFunState/1 -> Compare To Constant/1

== system TosAeb/AEB_Objsel/TA_Processor/PreProcessor/ObjectPrecessor/TAObjInfoCalc/For Each Subsystem/Info_Check1/Basic_Info_check/VxCorrect/Subsystem3/Subsystem1 ==
  Vx_CorrectFlg : Inport
  Fus_ObjVx_Single : Inport  Port=2
  Constant : Constant  Value=P_TA_ObjVxLowPassFactor
  LowPassFilter : Ta_Library/LowPassFilter  SystemSampleTime=-1  VariantActivationTime=update diagram  Latency=0  AutoFrameSizeCalculation=off
  Switch : Switch  Criteria=u2 ~= 0
  Fus_ObjVx : Outport
  wires:
  Switch/1 -> Fus_ObjVx/1
  Fus_ObjVx_Single/1 -> Switch/3, LowPassFilter/2
  LowPassFilter/1 -> Switch/1
  Vx_CorrectFlg/1 -> Switch/2
  Constant/1 -> LowPassFilter/1

== system TosAeb/AEB_Objsel/TA_Processor/PreProcessor/ObjectPrecessor/TAObjInfoCalc/For Each Subsystem/Info_Check1/Basic_Info_check/VxCorrect/Subsystem3/Subsystem2 ==
  Fus_ObjDx_Single : Inport
  Veh_AEBFunState : Inport  Port=2
  Fus_ObjVxStd : Inport  Port=3
  Fus_ObjVx_Single : Inport  Port=4
  Compare To Constant : simulink/Logic and Bit Operations/Compare To Constant  relop=>=  const=P_TA_ObjVxVarianceThredValue  OutDataTypeStr=boolean
  Compare To Constant1 : simulink/Logic and Bit Operations/Compare To Constant  relop=>=  const=P_TA_ObjVxSTDThredValue  OutDataTypeStr=boolean
  Data Type Conversion : DataTypeConversion
  Logical Operator : Logic  Operator=OR  Inputs=3  AllPortsSameDT=off  OutDataTypeStr=boolean
  Logical Operator1 : Logic  AllPortsSameDT=off  OutDataTypeStr=boolean
  Subsystem : Subsystem
  Subsystem1 : Subsystem
  Variance_Cal : Ta_Library/Variance_Cal  SystemSampleTime=-1  VariantActivationTime=update diagram  Latency=0  AutoFrameSizeCalculation=off
  Vx_CorrectFlg : Outport
  wires:
  Variance_Cal/1 -> Compare To Constant/1
  Data Type Conversion/1 -> Vx_CorrectFlg/1
  Logical Operator1/1 -> Data Type Conversion/1
  Subsystem/1(DisLong_flg) -> Logical Operator/2
  Logical Operator/1 -> Logical Operator1/1
  Veh_AEBFunState/1 -> Subsystem1/1(Veh_AEBFunState)
  Compare To Constant/1 -> Logical Operator/1
  Subsystem1/1(AEB_State) -> Logical Operator1/2
  Fus_ObjVxStd/1 -> Compare To Constant1/1
  Compare To Constant1/1 -> Logical Operator/3
  Fus_ObjVx_Single/1 -> Variance_Cal/1, Subsystem/1(Fus_ObjVx_Single)
  Fus_ObjDx_Single/1 -> Subsystem/2(Fus_ObjDx_Single)

== system TosAeb/AEB_Objsel/TA_Processor/PreProcessor/ObjectPrecessor/TAObjInfoCalc/For Each Subsystem/Info_Check1/Basic_Info_check/VyCorrect/Subsystem3/Subsystem1 ==
  Vy_CorrectFlg : Inport
  State_flg : Inport  Port=2
  Fus_ObjVy_Single : Inport  Port=3
  Constant : Constant  Value=P_TA_ObjVyLowPassFactor
  Constant1 : Constant  Value=0  OutDataTypeStr=single
  LowPassFilter : Ta_Library/LowPassFilter  SystemSampleTime=-1  VariantActivationTime=update diagram  Latency=0  AutoFrameSizeCalculation=off
  Switch : Switch  Criteria=u2 ~= 0
  Switch2 : Switch  Criteria=u2 ~= 0
  Fus_ObjVy : Outport
  wires:
  Switch2/1 -> Fus_ObjVy/1
  Constant1/1 -> Switch2/1
  State_flg/1 -> Switch2/2
  Vy_CorrectFlg/1 -> Switch/2
  Fus_ObjVy_Single/1 -> LowPassFilter/2, Switch/3
  Switch/1 -> Switch2/3
  LowPassFilter/1 -> Switch/1
  Constant/1 -> LowPassFilter/1

== system TosAeb/AEB_Objsel/TA_Processor/PreProcessor/ObjectPrecessor/TAObjInfoCalc/For Each Subsystem/Info_Check1/Basic_Info_check/VyCorrect/Subsystem3/Subsystem2 ==
  Fus_ObjDy_Single : Inport
  Veh_AEBFunState : Inport  Port=2
  Fus_TargetState : Inport  Port=3
  Fus_ObsVyStd : Inport  Port=4
  Veh_YawRate : Inport  Port=5
  Fus_ObjVy_Single : Inport  Port=6
  Compare To Constant : simulink/Logic and Bit Operations/Compare To Constant  relop=>=  const=P_TA_ObjVyVarianceThredValue  OutDataTypeStr=boolean
  Compare To Constant1 : simulink/Logic and Bit Operations/Compare To Constant  relop=>=  const=P_TA_ObjVySTDThredValue  OutDataTypeStr=boolean
  Data Type Conversion : DataTypeConversion
  Logical Operator : Logic  Operator=OR  Inputs=3  AllPortsSameDT=off  OutDataTypeStr=boolean
  Logical Operator1 : Logic  AllPortsSameDT=off  OutDataTypeStr=boolean
  Subsystem : Subsystem
  Subsystem1 : Subsystem
  Subsystem2 : Subsystem
  Variance_Cal : Ta_Library/Variance_Cal  SystemSampleTime=-1  VariantActivationTime=update diagram  Latency=0  AutoFrameSizeCalculation=off
  Vy_CorrectFlg : Outport
  State_flg : Outport  Port=2
  wires:
  Subsystem2/1(State_flg) -> State_flg/1
  Fus_TargetState/1 -> Subsystem2/1(Fus_TargetState)
  Subsystem1/1(AEB_State) -> Logical Operator1/2
  Compare To Constant/1 -> Logical Operator/1
  Veh_AEBFunState/1 -> Subsystem1/1(Veh_AEBFunState)
  Logical Operator/1 -> Logical Operator1/1
  Subsystem/1(DisLat_flg) -> Logical Operator/2
  Logical Operator1/1 -> Data Type Conversion/1
  Data Type Conversion/1 -> Vy_CorrectFlg/1
  Variance_Cal/1 -> Compare To Constant/1
  Compare To Constant1/1 -> Logical Operator/3
  Fus_ObsVyStd/1 -> Compare To Constant1/1
  Veh_YawRate/1 -> Subsystem2/2(Veh_YawRate)
  Fus_ObjVy_Single/1 -> Variance_Cal/1, Subsystem/1(Fus_ObjVy_Single)
  Fus_ObjDy_Single/1 -> Subsystem/2(Fus_ObjDy_Single)

== system TosAeb/AEB_Objsel/TA_Processor/PreProcessor/ObjectPrecessor/TAObjInfoCalc/For Each Subsystem/Info_Check1/Type_Process/Heading/Obj_AbsInfo/Subsystem ==
  TA_ObjInfo : Inport
  For_Number : Inport  Port=2
  VehSelfData : Inport  Port=3
  Add : Sum
  Add1 : Sum
  Atan2 : Trigonometry  Operator=atan2
  Bus Selector2 : BusSelector  OutputSignals=TA_ObjAccel,TA_Objvx,TA_Objvy,TA_ObjState
  Bus Selector3 : BusSelector  OutputSignals=VehSelf.Veh_LongSpd_mps,VehSelf.YawRate
  Compare To Constant : simulink/Logic and Bit Operations/Compare To Constant  relop===  const=0  OutDataTypeStr=boolean
  Compare To Constant1 : simulink/Logic and Bit Operations/Compare To Constant  relop===  const=1  OutDataTypeStr=boolean
  Constant : Constant  Value=pi/2  OutDataTypeStr=single
  Constant1 : Constant  Value=0  OutDataTypeStr=single
  Constant16 : Constant  Value=P_TA_PrmForVehRearAxleDisttoFrnt
  Constant2 : Constant  Value=0  OutDataTypeStr=single
  Index Vector{,1..3} (4 alike) : MultiPortSwitch  Inputs=1
  Logical Operator : Logic  Operator=OR  AllPortsSameDT=off  OutDataTypeStr=boolean
  Product : Product
  Switch : Switch  Criteria=u2 > Threshold
  Switch1 : Switch  Criteria=u2 > Threshold
  <TA_ObjAccel> : Outport
  Obj_AbsVy : Outport  Port=2
  InputAngle : Outport  Port=3
  MaxAngle : Outport  Port=4
  wires:
  Add/1 -> Atan2/2
  Bus Selector3/2 -> Product/1  "<YawRate>"
  Add1/1 -> Switch1/3, Atan2/1
  Bus Selector2/2 -> Index Vector1/2  "<TA_Objvx>"
  TA_ObjInfo/1 -> Bus Selector2/1
  Constant16/1 -> Product/2
  Product/1 -> Add1/2
  Bus Selector2/3 -> Index Vector2/2  "<TA_Objvy>"
  Bus Selector3/1 -> Add/2  "<Veh_LongSpd_mps>"
  VehSelfData/1 -> Bus Selector3/1
  Bus Selector2/1 -> Index Vector/2  "<TA_ObjAccel>"
  Constant/1 -> MaxAngle/1
  Index Vector/1 -> <TA_ObjAccel>/1
  For_Number/1 -> [K], Index Vector/1
  Index Vector1/1 -> Add/1
  Index Vector2/1 -> Add1/1
  [K] -> Index Vector1/1
  [K] -> Index Vector2/1
  Bus Selector2/4 -> [state]  "<TA_ObjState>"
  Index Vector3/1 -> Compare To Constant1/1, Compare To Constant/1
  Compare To Constant/1 -> Logical Operator/1
  Compare To Constant1/1 -> Logical Operator/2
  Logical Operator/1 -> Switch/2, Switch1/2
  Constant1/1 -> Switch/1
  Atan2/1 -> Switch/3
  Switch/1 -> InputAngle/1
  Switch1/1 -> Obj_AbsVy/1
  Constant2/1 -> Switch1/1
  [state] -> Index Vector3/2
  [K] -> Index Vector3/1

== system TosAeb/AEB_Objsel/TA_Processor/PreProcessor/ObjectPrecessor/TAObjInfoCalc/For Each Subsystem/Info_Check1/Type_Process/Heading/Obj_AbsInfo/Subsystem1 ==
  TA_ObjAccel : Inport
  Obj_AbsVy : Inport  Port=2
  MappedAngle : Inport  Port=3
  Abs : Abs
  Compare To Constant : simulink/Logic and Bit Operations/Compare To Constant  relop===  const=pi/2  OutDataTypeStr=boolean
  Constant : Constant  Value=0.001  OutDataTypeStr=single
  Product : Product
  Signal Conversion : SignalConversion  OverrideOpt=off
  Sin : Trigonometry  Operator=tan
  Switch : Switch  Criteria=u2 > Threshold
  Obj_AbsAccly : Outport
  Obj_AbsSpdy : Outport  Port=2
  wires:
  MappedAngle/1 -> Switch/3, Abs/1
  TA_ObjAccel/1 -> Product/1
  Product/1 -> Obj_AbsAccly/1
  Obj_AbsVy/1 -> Signal Conversion/1
  Signal Conversion/1 -> Obj_AbsSpdy/1
  Compare To Constant/1 -> Switch/2
  Abs/1 -> Compare To Constant/1
  Constant/1 -> Switch/1
  Switch/1 -> Sin/1
  Sin/1 -> Product/2

== system TosAeb/FcwAebJa_Step_sys/ALG_AEBSystem/ALG_AEBController/AEB_FuncCondCalcApp/CycFunctionCondCalc/cycAEBfullSuppAborCond/cycAEBfullSuppCondCalc/cycAEBfullSituationRelSuppCondCalc/LatDistCond4fullBrakeCalc/LatDistThresCalc/LateralDistanceThresHMISettingEarly ==
  HMISettingEarlyOrMiddle : ActionPort  ActionPortLabel=case [ HMISettingEarly HMISettingMiddle ]:
  Constant : Constant  Value=P_AEB_Func_cycAEBfullDyLatBoundHmiEarlyMid  OutDataTypeStr=single
  latDistThres : Outport
  wires:
  Constant/1 -> latDistThres/1

== system TosAeb/FcwAebJa_Step_sys/ALG_AEBSystem/ALG_AEBController/AEB_FuncCondCalcApp/CycFunctionCondCalc/cycAEBfullSuppAborCond/cycAEBfullSuppCondCalc/cycAEBfullSituationRelSuppCondCalc/LatDistCond4fullBrakeCalc/LatDistThresCalc/LateralDistanceThresHMISettingLate ==
  vy : Inport
  HMISettingLate : ActionPort  ActionPortLabel=default:
  Abs : Abs
  LateralDistanceThresLookupTable : Lookup_n-D  NumberOfTableDimensions=1  BreakpointsForDimension1=P_AEB_Func_cycAEBfullObjVyAbsLatBoundHmiLateX  BeginIndexSearchUsingPreviousIndexResult=on  Table=P_AEB_Func_cycAEBfullDyLatBoundHmiLateY  InternalRulePriority=Speed  RndMeth=Simplest  InputSameDT=on
  latDistThres : Outport
  wires:
  Abs/1 -> LateralDistanceThresLookupTable/1
  LateralDistanceThresLookupTable/1 -> latDistThres/1
  vy/1 -> Abs/1

== system TosAeb/FcwAebJa_Step_sys/ALG_AEBSystem/ALG_AEBController/AEB_FuncCondCalcApp/CycFunctionCondCalc/cycAEBpartSuppAborCond/cycAEBpartSuppCondCalc/cycAEBpartSituationRelActiveCondCalc/LatDistCond4PartBrakeCalc/LatDistThresCalc/LateralDistanceThresHMISettingEarly ==
  vyCorr : Inport
  HMISettingEarlyOrMiddle : ActionPort  ActionPortLabel=case [ HMISettingEarly HMISettingMiddle ]:
  LateralDistanceThresLookupTable : Lookup_n-D  NumberOfTableDimensions=1  BreakpointsForDimension1=P_AEB_Func_cycAEBparObjVyAbsLatBoundHmiEarlyMidX  BeginIndexSearchUsingPreviousIndexResult=on  Table=P_AEB_Func_cycAEBpartDyLatBoundHmiEarlyMidY  InternalRulePriority=Speed  RndMeth=Simplest  InputSameDT=on
  latDistThres : Outport
  wires:
  vyCorr/1 -> LateralDistanceThresLookupTable/1
  LateralDistanceThresLookupTable/1 -> latDistThres/1
